simulink model "v01_aa_rii_16r4t11f_ver124sa"  (Simulink 7.6)
settings: Solver=ode45  StartTime=0.0  StopTime=128000  RelTol=1e-3
undefined symbols (not defined in the file or in its folder): sfp
libraries: xbsIndex_r4 x27287, monroe_library x154, xbsReplacements_r4 x78, casper_library_misc x33, simulink x32, dspsigops x32, xps_library x24
(part 4 of 178)

== system v01_aa_rii_16r4t11f_ver124sa/cram4 ==
  In1 : Inport
  In2 : Inport  Port=2
  In3 : Inport  Port=3
  In4 : Inport  Port=4
  In5 : Inport  Port=5
  In6 : Inport  Port=6
  In7 : Inport  Port=7
  In8 : Inport  Port=8
  In9 : Inport  Port=9
  In10 : Inport  Port=10
  In11 : Inport  Port=11
  In12 : Inport  Port=12
  In13 : Inport  Port=13
  In14 : Inport  Port=14
  In15 : Inport  Port=15
  In16 : Inport  Port=16
  In17 : Inport  Port=17
  In18 : Inport  Port=18
  In19 : Inport  Port=19
  In20 : Inport  Port=20
  In21 : Inport  Port=21
  In22 : Inport  Port=22
  In23 : Inport  Port=23
  In24 : Inport  Port=24
  In25 : Inport  Port=25
  In26 : Inport  Port=26
  In27 : Inport  Port=27
  In28 : Inport  Port=28
  In29 : Inport  Port=29
  In30 : Inport  Port=30
  In31 : Inport  Port=31
  In32 : Inport  Port=32
  Concat : xbsIndex_r4/Concat  num_inputs=32  dbl_ovrd=off  block_type=concat
      settings: block_version=10.1.2
  Reinterp{1..32} (32 alike) : xbsIndex_r4/Reinterpret  force_arith_type=on  arith_type=Unsigned  force_bin_pt=on  bin_pt=0  block_type=reinterpret
      Reinterp1 settings: infoedit="Changes signal type without altering the binary representation.   You can changed the signal between signed and unsigned, and relocate the binary point.<P><P>Hardware notes: In hardware this block costs nothing.<P><P>Example:  Suppose the input is 6 bits wide, signed, with 2 fractional bits, and the output is forced to unsigned with 0 fractional bits.  Then an input of -2.0 (1110.00 in binary 2's complement) becomes an output of 56 (111000 in binary)."  block_version=10.1.2
      Reinterp10 settings: infoedit="Changes signal type without altering the binary representation.   You can changed the signal between signed and unsigned, and relocate the binary point.<br><br>Hardware notes: In hardware this block costs nothing.<br><br>Example:  Suppose the input is 6 bits wide, signed, with 2 fractional bits, and the output is forced to unsigned with 0 fractional bits.  Then an input of -2.0 (1110.00 in binary 2's complement) becomes an output of 56 (111000 in binary)."
      Reinterp11 settings: infoedit="Changes signal type without altering the binary representation.   You can changed the signal between signed and unsigned, and relocate the binary point.<br><br>Hardware notes: In hardware this block costs nothing.<br><br>Example:  Suppose the input is 6 bits wide, signed, with 2 fractional bits, and the output is forced to unsigned with 0 fractional bits.  Then an input of -2.0 (1110.00 in binary 2's complement) becomes an output of 56 (111000 in binary)."
      Reinterp12 settings: infoedit="Changes signal type without altering the binary representation.   You can changed the signal between signed and unsigned, and relocate the binary point.<br><br>Hardware notes: In hardware this block costs nothing.<br><br>Example:  Suppose the input is 6 bits wide, signed, with 2 fractional bits, and the output is forced to unsigned with 0 fractional bits.  Then an input of -2.0 (1110.00 in binary 2's complement) becomes an output of 56 (111000 in binary)."
      Reinterp13 settings: infoedit="Changes signal type without altering the binary representation.   You can changed the signal between signed and unsigned, and relocate the binary point.<br><br>Hardware notes: In hardware this block costs nothing.<br><br>Example:  Suppose the input is 6 bits wide, signed, with 2 fractional bits, and the output is forced to unsigned with 0 fractional bits.  Then an input of -2.0 (1110.00 in binary 2's complement) becomes an output of 56 (111000 in binary)."
      Reinterp14 settings: infoedit="Changes signal type without altering the binary representation.   You can changed the signal between signed and unsigned, and relocate the binary point.<br><br>Hardware notes: In hardware this block costs nothing.<br><br>Example:  Suppose the input is 6 bits wide, signed, with 2 fractional bits, and the output is forced to unsigned with 0 fractional bits.  Then an input of -2.0 (1110.00 in binary 2's complement) becomes an output of 56 (111000 in binary)."
      Reinterp15 settings: infoedit="Changes signal type without altering the binary representation.   You can changed the signal between signed and unsigned, and relocate the binary point.<br><br>Hardware notes: In hardware this block costs nothing.<br><br>Example:  Suppose the input is 6 bits wide, signed, with 2 fractional bits, and the output is forced to unsigned with 0 fractional bits.  Then an input of -2.0 (1110.00 in binary 2's complement) becomes an output of 56 (111000 in binary)."
      Reinterp16 settings: infoedit="Changes signal type without altering the binary representation.   You can changed the signal between signed and unsigned, and relocate the binary point.<br><br>Hardware notes: In hardware this block costs nothing.<br><br>Example:  Suppose the input is 6 bits wide, signed, with 2 fractional bits, and the output is forced to unsigned with 0 fractional bits.  Then an input of -2.0 (1110.00 in binary 2's complement) becomes an output of 56 (111000 in binary)."
      Reinterp2 settings: infoedit="Changes signal type without altering the binary representation.   You can changed the signal between signed and unsigned, and relocate the binary point.<P><P>Hardware notes: In hardware this block costs nothing.<P><P>Example:  Suppose the input is 6 bits wide, signed, with 2 fractional bits, and the output is forced to unsigned with 0 fractional bits.  Then an input of -2.0 (1110.00 in binary 2's complement) becomes an output of 56 (111000 in binary)."  block_version=10.1.2
      Reinterp3 settings: infoedit="Changes signal type without altering the binary representation.   You can changed the signal between signed and unsigned, and relocate the binary point.<br><br>Hardware notes: In hardware this block costs nothing.<br><br>Example:  Suppose the input is 6 bits wide, signed, with 2 fractional bits, and the output is forced to unsigned with 0 fractional bits.  Then an input of -2.0 (1110.00 in binary 2's complement) becomes an output of 56 (111000 in binary)."
      Reinterp4 settings: infoedit="Changes signal type without altering the binary representation.   You can changed the signal between signed and unsigned, and relocate the binary point.<br><br>Hardware notes: In hardware this block costs nothing.<br><br>Example:  Suppose the input is 6 bits wide, signed, with 2 fractional bits, and the output is forced to unsigned with 0 fractional bits.  Then an input of -2.0 (1110.00 in binary 2's complement) becomes an output of 56 (111000 in binary)."
      Reinterp5 settings: infoedit="Changes signal type without altering the binary representation.   You can changed the signal between signed and unsigned, and relocate the binary point.<br><br>Hardware notes: In hardware this block costs nothing.<br><br>Example:  Suppose the input is 6 bits wide, signed, with 2 fractional bits, and the output is forced to unsigned with 0 fractional bits.  Then an input of -2.0 (1110.00 in binary 2's complement) becomes an output of 56 (111000 in binary)."
      Reinterp6 settings: infoedit="Changes signal type without altering the binary representation.   You can changed the signal between signed and unsigned, and relocate the binary point.<br><br>Hardware notes: In hardware this block costs nothing.<br><br>Example:  Suppose the input is 6 bits wide, signed, with 2 fractional bits, and the output is forced to unsigned with 0 fractional bits.  Then an input of -2.0 (1110.00 in binary 2's complement) becomes an output of 56 (111000 in binary)."
      Reinterp7 settings: infoedit="Changes signal type without altering the binary representation.   You can changed the signal between signed and unsigned, and relocate the binary point.<br><br>Hardware notes: In hardware this block costs nothing.<br><br>Example:  Suppose the input is 6 bits wide, signed, with 2 fractional bits, and the output is forced to unsigned with 0 fractional bits.  Then an input of -2.0 (1110.00 in binary 2's complement) becomes an output of 56 (111000 in binary)."
      Reinterp8 settings: infoedit="Changes signal type without altering the binary representation.   You can changed the signal between signed and unsigned, and relocate the binary point.<br><br>Hardware notes: In hardware this block costs nothing.<br><br>Example:  Suppose the input is 6 bits wide, signed, with 2 fractional bits, and the output is forced to unsigned with 0 fractional bits.  Then an input of -2.0 (1110.00 in binary 2's complement) becomes an output of 56 (111000 in binary)."
      Reinterp9 settings: infoedit="Changes signal type without altering the binary representation.   You can changed the signal between signed and unsigned, and relocate the binary point.<br><br>Hardware notes: In hardware this block costs nothing.<br><br>Example:  Suppose the input is 6 bits wide, signed, with 2 fractional bits, and the output is forced to unsigned with 0 fractional bits.  Then an input of -2.0 (1110.00 in binary 2's complement) becomes an output of 56 (111000 in binary)."
  Out : Outport
  wires:
  Concat/1 -> Out/1
  In1/1 -> Reinterp1/1
  Reinterp1/1 -> Concat/1
  In2/1 -> Reinterp2/1
  Reinterp2/1 -> Concat/2
  In3/1 -> Reinterp3/1
  Reinterp3/1 -> Concat/3
  In4/1 -> Reinterp4/1
  Reinterp4/1 -> Concat/4
  In5/1 -> Reinterp5/1
  Reinterp5/1 -> Concat/5
  In6/1 -> Reinterp6/1
  Reinterp6/1 -> Concat/6
  In7/1 -> Reinterp7/1
  Reinterp7/1 -> Concat/7
  In8/1 -> Reinterp8/1
  Reinterp8/1 -> Concat/8
  In9/1 -> Reinterp9/1
  Reinterp9/1 -> Concat/9
  In10/1 -> Reinterp10/1
  Reinterp10/1 -> Concat/10
  In11/1 -> Reinterp11/1
  Reinterp11/1 -> Concat/11
  In12/1 -> Reinterp12/1
  Reinterp12/1 -> Concat/12
  In13/1 -> Reinterp13/1
  Reinterp13/1 -> Concat/13
  In14/1 -> Reinterp14/1
  Reinterp14/1 -> Concat/14
  In15/1 -> Reinterp15/1
  Reinterp15/1 -> Concat/15
  In16/1 -> Reinterp16/1
  Reinterp16/1 -> Concat/16
  In17/1 -> Reinterp17/1
  Reinterp17/1 -> Concat/17
  In18/1 -> Reinterp18/1
  Reinterp18/1 -> Concat/18
  In19/1 -> Reinterp19/1
  Reinterp19/1 -> Concat/19
  In20/1 -> Reinterp20/1
  Reinterp20/1 -> Concat/20
  In21/1 -> Reinterp21/1
  Reinterp21/1 -> Concat/21
  In22/1 -> Reinterp22/1
  Reinterp22/1 -> Concat/22
  In23/1 -> Reinterp23/1
  Reinterp23/1 -> Concat/23
  In24/1 -> Reinterp24/1
  Reinterp24/1 -> Concat/24
  In25/1 -> Reinterp25/1
  Reinterp25/1 -> Concat/25
  In26/1 -> Reinterp26/1
  Reinterp26/1 -> Concat/26
  In27/1 -> Reinterp27/1
  Reinterp27/1 -> Concat/27
  In28/1 -> Reinterp28/1
  Reinterp28/1 -> Concat/28
  In29/1 -> Reinterp29/1
  Reinterp29/1 -> Concat/29
  In30/1 -> Reinterp30/1
  Reinterp30/1 -> Concat/30
  In31/1 -> Reinterp31/1
  Reinterp31/1 -> Concat/31
  In32/1 -> Reinterp32/1
  Reinterp32/1 -> Concat/32

== system v01_aa_rii_16r4t11f_ver124sa/debug ==
  note: Generate stop
      | when in circular
      | capture mode
  note: Delay start
      | of capture
  note: ctrl:
      | bit0: Put a posedge here
      |         to enable a new capture.
      | bit1: ignore external trigger
      |         and trigger immediately.
      | bit2: ignore external write
      |         enable and capture on
      |         every FPGA clock.
      | bit3: continuously capture
      |         data until we get an
      |         external stop command.
  note: Offset in vector
      | from first element
      | captured, used
      | during circular capture
  note: Number of
      | bytes in
      | buffer and
      | done bit
  note: Number of valid
      | data samples to
      | skip after trigger
      | before starting
      | capture
  note: Value on vin
      | aligned with
      | first sample on
      | din captured
  din : Inport
  we : Inport  Port=2
  trig : Inport  Port=3
  add_del : xbsIndex_r4/Delay  rst=off  infoeditControl=Selection of Reset will increase slice count due to use of real FFs and instead of SRLs  en=off  latency=1  dbl_ovrd=off  reg_retiming=off  block_type=delay
  add_gen : Subsystem  AncestorBlock=casper_library_scopes/snapshot/add_gen  mask{counter_size=14+2+1, increment=4, nsamples=14, burst_size=0, use_dsp48=on}
      mask prompts: counter_size "Address counter size (2^?)"; increment "Address counter increment"; nsamples "Number of samples (2^?)"; burst_size "Burst size (2^?)"; use_dsp48 "dsp48 counter/s"
      mask init:
      | set_param(gcb,'LinkStatus','inactive');
      | if strcmp(use_dsp48,'on'),
      |     set_param([gcb,'/add_gen'],'implementation','DSP48');
      | else
      |     set_param([gcb,'/add_gen'],'implementation','Fabric');
      | end
  basic_ctrl : Subsystem  AncestorBlock=casper_library_scopes/snapshot/basic_ctrl  mask{dram=off, data_width=32}
      mask prompts: data_width "data width"
      mask init:
      | set_param(gcb,'LinkStatus','inactive');
      | set_param([gcb,'/dram_munge'], 'dram', dram);
  bram : Subsystem  Tag=xps:bram  AncestorBlock=xps_library/Shared BRAM  mask{arith_type=Unsigned, addr_width=14, data_width=32, data_bin_pt=0, init_vals=[0:2^10-1], sample_rate=1}
      mask prompts: arith_type "Output Data Type"; addr_width "Address width"; data_width "Data Width"; data_bin_pt "Data Binary Point"; init_vals "Initial 32-bit values (simulation only)"; sample_rate "Sample rate"
      mask init:
      | shared_bram_mask;
      mask StyleString: popup(Unsigned|Signed  (2's comp)),edit,popup(8|16|32|64|128),edit,edit,edit
  cast : xbsIndex_r4/Convert  gui_display_data_type=Fixed-point  arith_type=Unsigned  n_bits=32  bin_pt=0  float_type=Single  exp_bits=8  fraction_bits=24  quantization=Truncate  overflow=Wrap  en=off  latency=0  dbl_ovrd=off  pipeline=off  block_type=convert
  const0 : Constant  Value=0
  ctrl : xps_library/software register  Tag=xps:sw_reg  SystemSampleTime=-1  io_dir=From Processor  arith_type=Unsigned  bitwidth=32  bin_pt=0  sample_period=1  gw_name=v01_aa_rii_16r4t11f_ver122sa_debug_ctrl_user_data_out
  dat_del : xbsIndex_r4/Delay  rst=off  infoeditControl=Selection of Reset will increase slice count due to use of real FFs and instead of SRLs  en=off  latency=1  dbl_ovrd=off  reg_retiming=off  block_type=delay
  g_tr_en_cnt : Terminator
  gbram : Terminator
  gstatus : Terminator
  gval : Terminator
  never : xbsIndex_r4/Constant  const=0  gui_display_data_type=Fixed-point  arith_type=Boolean  n_bits=16  bin_pt=14  preci_type=Single  exp_width=8  frac_width=24  explicit_period=on  period=1  equ=P=C  opselect=C  inp2=PCIN>>17  opr=+  inp1=P  carry=CIN  dbl_ovrd=off  block_type=constant
  ri : xbsIndex_r4/Reinterpret  force_arith_type=on  arith_type=Unsigned  force_bin_pt=on  bin_pt=0  block_type=reinterpret
  status : xps_library/software register  Tag=xps:sw_reg  SystemSampleTime=-1  io_dir=To Processor  arith_type=Unsigned  bitwidth=32  bin_pt=0  sample_period=1  gw_name=v01_aa_rii_16r4t11f_ver122sa_debug_status_user_data_in
  vin_const : xbsIndex_r4/Constant  const=0  gui_display_data_type=Fixed-point  arith_type=Boolean  n_bits=16  bin_pt=14  preci_type=Single  exp_width=8  frac_width=24  explicit_period=on  period=1  equ=P=C  opselect=C  inp2=PCIN>>17  opr=+  inp1=P  carry=CIN  dbl_ovrd=off  block_type=constant
  we_del : xbsIndex_r4/Delay  rst=off  infoeditControl=Selection of Reset will increase slice count due to use of real FFs and instead of SRLs  en=off  latency=1  dbl_ovrd=off  reg_retiming=off  block_type=delay
  wires:
  din/1 -> ri/1
  ri/1 -> cast/1
  cast/1 -> basic_ctrl/2(din)
  we/1 -> basic_ctrl/3(we)
  trig/1 -> basic_ctrl/4(trig)
  never/1 -> basic_ctrl/6(stopi)
  const0/1 -> ctrl/1
  ctrl/1 -> basic_ctrl/5(ctrl)
  basic_ctrl/1(vout) -> add_gen/1(vin)
  basic_ctrl/2(dout) -> add_gen/2(din)
  basic_ctrl/3(we_o) -> add_gen/3(we)
  basic_ctrl/4(go) -> add_gen/4(go)
  basic_ctrl/5(stop) -> add_gen/5(cont)
  basic_ctrl/6(init) -> add_gen/6(init)
  add_gen/5(status) -> status/1
  status/1 -> gstatus/1
  vin_const/1 -> basic_ctrl/1(vin)
  add_gen/1(vout) -> gval/1
  add_gen/6(en_cnt) -> g_tr_en_cnt/1
  add_gen/2(add) -> add_del/1
  add_gen/3(dout) -> dat_del/1
  add_gen/4(we_o) -> we_del/1
  add_del/1 -> bram/1(addr)
  dat_del/1 -> bram/2(data_in)
  we_del/1 -> bram/3(we)
  bram/1(data_out) -> gbram/1

== system v01_aa_rii_16r4t11f_ver124sa/fft_wideband_real ==
  sync : Inport
  shift : Inport  Port=2
  din_0 : Inport  Port=3
  din_1 : Inport  Port=4
  din_2 : Inport  Port=5
  din_3 : Inport  Port=6
  din_4 : Inport  Port=7
  din_5 : Inport  Port=8
  din_6 : Inport  Port=9
  din_7 : Inport  Port=10
  din_8 : Inport  Port=11
  din_9 : Inport  Port=12
  din_10 : Inport  Port=13
  din_11 : Inport  Port=14
  din_12 : Inport  Port=15
  din_13 : Inport  Port=16
  din_14 : Inport  Port=17
  din_15 : Inport  Port=18
  fft_biplex_real_4x0 : Subsystem
      settings: AttributesFormatString=|
      | Virtex5
      | 7 stages
      | [18,18]
      | Truncate
      | Wrap
      | 0 0 0 0 0 0 0 0 0 0 0 0 0 0 0 0 0 0 0
  fft_biplex_real_4x1 : Subsystem
      settings: AttributesFormatString=|
      | Virtex5
      | 7 stages
      | [18,18]
      | Truncate
      | Wrap
      | 0 0 0 0 0 0 0 0 0 0 0 0 0 0 0 0 0 0 0
  fft_biplex_real_4x2 : Subsystem
      settings: AttributesFormatString=|
      | Virtex5
      | 7 stages
      | [18,18]
      | Truncate
      | Wrap
      | 0 0 0 0 0 0 0 0 0 0 0 0 0 0 0 0 0 0 0
  fft_biplex_real_4x3 : Subsystem
      settings: AttributesFormatString=|
      | Virtex5
      | 7 stages
      | [18,18]
      | Truncate
      | Wrap
      | 0 0 0 0 0 0 0 0 0 0 0 0 0 0 0 0 0 0 0
  fft_direct : Subsystem
      settings: AttributesFormatString=|
      | Virtex5
      | stages [8   9  10  11] of 11
      | [18,18]
      | Truncate
      | Wrap
      | 0 0 0 0 0 0 0 0 0
  fft_unscrambler : Subsystem
      settings: AttributesFormatString=FFTSize=10, n_inputs=3
  in_del_4x0pol1 : Subsystem
      settings: AttributesFormatString=Latency = 0
  in_del_4x0pol2 : Subsystem
      settings: AttributesFormatString=Latency = 0
  in_del_4x0pol3 : Subsystem
      settings: AttributesFormatString=Latency = 0
  in_del_4x0pol4 : Subsystem
      settings: AttributesFormatString=Latency = 0
  in_del_4x1pol1 : Subsystem
      settings: AttributesFormatString=Latency = 0
  in_del_4x1pol2 : Subsystem
      settings: AttributesFormatString=Latency = 0
  in_del_4x1pol3 : Subsystem
      settings: AttributesFormatString=Latency = 0
  in_del_4x1pol4 : Subsystem
      settings: AttributesFormatString=Latency = 0
  in_del_4x2pol1 : Subsystem
      settings: AttributesFormatString=Latency = 0
  in_del_4x2pol2 : Subsystem
      settings: AttributesFormatString=Latency = 0
  in_del_4x2pol3 : Subsystem
      settings: AttributesFormatString=Latency = 0
  in_del_4x2pol4 : Subsystem
      settings: AttributesFormatString=Latency = 0
  in_del_4x3pol1 : Subsystem
      settings: AttributesFormatString=Latency = 0
  in_del_4x3pol2 : Subsystem
      settings: AttributesFormatString=Latency = 0
  in_del_4x3pol3 : Subsystem
      settings: AttributesFormatString=Latency = 0
  in_del_4x3pol4 : Subsystem
      settings: AttributesFormatString=Latency = 0
  in_del_sync_4x0 : Subsystem
      settings: AttributesFormatString=Latency = 0
  in_del_sync_4x1 : Subsystem
      settings: AttributesFormatString=Latency = 0
  in_del_sync_4x2 : Subsystem
      settings: AttributesFormatString=Latency = 0
  in_del_sync_4x3 : Subsystem
      settings: AttributesFormatString=Latency = 0
  of_det : xbsIndex_r4/Logical  logical_function=OR  inputs=5  en=off  latency=1  precision=Full  arith_type=Unsigned  n_bits=16  bin_pt=0  align_bp=on  dbl_ovrd=off  block_type=logical
  out_del_4x0pol1 : Subsystem
      settings: AttributesFormatString=Latency = 0
  out_del_4x0pol2 : Subsystem
      settings: AttributesFormatString=Latency = 0
  out_del_4x0pol3 : Subsystem
      settings: AttributesFormatString=Latency = 0
  out_del_4x0pol4 : Subsystem
      settings: AttributesFormatString=Latency = 0
  out_del_4x1pol1 : Subsystem
      settings: AttributesFormatString=Latency = 0
  out_del_4x1pol2 : Subsystem
      settings: AttributesFormatString=Latency = 0
  out_del_4x1pol3 : Subsystem
      settings: AttributesFormatString=Latency = 0
  out_del_4x1pol4 : Subsystem
      settings: AttributesFormatString=Latency = 0
  out_del_4x2pol1 : Subsystem
      settings: AttributesFormatString=Latency = 0
  out_del_4x2pol2 : Subsystem
      settings: AttributesFormatString=Latency = 0
  out_del_4x2pol3 : Subsystem
      settings: AttributesFormatString=Latency = 0
  out_del_4x2pol4 : Subsystem
      settings: AttributesFormatString=Latency = 0
  out_del_4x3pol1 : Subsystem
      settings: AttributesFormatString=Latency = 0
  out_del_4x3pol2 : Subsystem
      settings: AttributesFormatString=Latency = 0
  out_del_4x3pol3 : Subsystem
      settings: AttributesFormatString=Latency = 0
  out_del_4x3pol4 : Subsystem
      settings: AttributesFormatString=Latency = 0
  out_del_sync_4x0 : Subsystem
      settings: AttributesFormatString=Latency = 0
  shift_slice : xbsIndex_r4/Slice  nbits=4  boolean_output=off  mode=Lower Bit Location + Width  bit1=0  base1=MSB of Input  bit0=7  base0=LSB of Input  dbl_ovrd=off  block_type=slice
  sync_out : Outport
  dout0 : Outport  Port=2
  dout1 : Outport  Port=3
  dout2 : Outport  Port=4
  dout3 : Outport  Port=5
  dout4 : Outport  Port=6
  dout5 : Outport  Port=7
  dout6 : Outport  Port=8
  dout7 : Outport  Port=9
  of : Outport  Port=10
  wires:
  shift/1 -> fft_biplex_real_4x3/2(shift), fft_biplex_real_4x2/2(shift), fft_biplex_real_4x1/2(shift), fft_biplex_real_4x0/2(shift), shift_slice/1
  sync/1 -> in_del_sync_4x3/1(d), in_del_sync_4x2/1(d), in_del_sync_4x1/1(d), in_del_sync_4x0/1(d)
  of_det/1 -> of/1
  fft_direct/18(of) -> of_det/5
  fft_biplex_real_4x3/6(of) -> of_det/4
  fft_biplex_real_4x2/6(of) -> of_det/3
  fft_biplex_real_4x1/6(of) -> of_det/2
  fft_biplex_real_4x0/6(of) -> of_det/1
  fft_unscrambler/9(Out8) -> dout7/1
  fft_unscrambler/8(Out7) -> dout6/1
  fft_unscrambler/7(Out6) -> dout5/1
  fft_unscrambler/6(Out5) -> dout4/1
  fft_unscrambler/5(Out4) -> dout3/1
  fft_unscrambler/4(Out3) -> dout2/1
  fft_unscrambler/3(Out2) -> dout1/1
  fft_unscrambler/2(Out1) -> dout0/1
  fft_unscrambler/1(sync_out) -> sync_out/1
  fft_direct/9(dout_7) -> fft_unscrambler/9(In8)
  fft_direct/8(dout_6) -> fft_unscrambler/8(In7)
  fft_direct/7(dout_5) -> fft_unscrambler/7(In6)
  fft_direct/6(dout_4) -> fft_unscrambler/6(In5)
  fft_direct/5(dout_3) -> fft_unscrambler/5(In4)
  fft_direct/4(dout_2) -> fft_unscrambler/4(In3)
  fft_direct/3(dout_1) -> fft_unscrambler/3(In2)
  fft_direct/2(dout_0) -> fft_unscrambler/2(In1)
  fft_direct/1(sync_out) -> fft_unscrambler/1(sync)
  out_del_4x3pol4/1(q) -> fft_direct/18(din_15)
  out_del_4x3pol3/1(q) -> fft_direct/17(din_14)
  out_del_4x3pol2/1(q) -> fft_direct/16(din_13)
  out_del_4x3pol1/1(q) -> fft_direct/15(din_12)
  out_del_4x2pol4/1(q) -> fft_direct/14(din_11)
  out_del_4x2pol3/1(q) -> fft_direct/13(din_10)
  out_del_4x2pol2/1(q) -> fft_direct/12(din_9)
  out_del_4x2pol1/1(q) -> fft_direct/11(din_8)
  out_del_4x1pol4/1(q) -> fft_direct/10(din_7)
  out_del_4x1pol3/1(q) -> fft_direct/9(din_6)
  out_del_4x1pol2/1(q) -> fft_direct/8(din_5)
  out_del_4x1pol1/1(q) -> fft_direct/7(din_4)
  out_del_4x0pol4/1(q) -> fft_direct/6(din_3)
  out_del_4x0pol3/1(q) -> fft_direct/5(din_2)
  out_del_4x0pol2/1(q) -> fft_direct/4(din_1)
  out_del_4x0pol1/1(q) -> fft_direct/3(din_0)
  shift_slice/1 -> fft_direct/2(shift)
  out_del_sync_4x0/1(q) -> fft_direct/1(sync)
  fft_biplex_real_4x3/5(pol4_out) -> out_del_4x3pol4/1(d)
  fft_biplex_real_4x3/4(pol3_out) -> out_del_4x3pol3/1(d)
  fft_biplex_real_4x3/3(pol2_out) -> out_del_4x3pol2/1(d)
  fft_biplex_real_4x3/2(pol1_out) -> out_del_4x3pol1/1(d)
  in_del_4x3pol4/1(q) -> fft_biplex_real_4x3/6(pol4)
  in_del_4x3pol3/1(q) -> fft_biplex_real_4x3/5(pol3)
  in_del_4x3pol2/1(q) -> fft_biplex_real_4x3/4(pol2)
  in_del_4x3pol1/1(q) -> fft_biplex_real_4x3/3(pol1)
  in_del_sync_4x3/1(q) -> fft_biplex_real_4x3/1(sync)
  din_15/1 -> in_del_4x3pol4/1(d)
  din_14/1 -> in_del_4x3pol3/1(d)
  din_13/1 -> in_del_4x3pol2/1(d)
  din_12/1 -> in_del_4x3pol1/1(d)
  fft_biplex_real_4x2/5(pol4_out) -> out_del_4x2pol4/1(d)
  fft_biplex_real_4x2/4(pol3_out) -> out_del_4x2pol3/1(d)
  fft_biplex_real_4x2/3(pol2_out) -> out_del_4x2pol2/1(d)
  fft_biplex_real_4x2/2(pol1_out) -> out_del_4x2pol1/1(d)
  in_del_4x2pol4/1(q) -> fft_biplex_real_4x2/6(pol4)
  in_del_4x2pol3/1(q) -> fft_biplex_real_4x2/5(pol3)
  in_del_4x2pol2/1(q) -> fft_biplex_real_4x2/4(pol2)
  in_del_4x2pol1/1(q) -> fft_biplex_real_4x2/3(pol1)
  in_del_sync_4x2/1(q) -> fft_biplex_real_4x2/1(sync)
  din_11/1 -> in_del_4x2pol4/1(d)
  din_10/1 -> in_del_4x2pol3/1(d)
  din_9/1 -> in_del_4x2pol2/1(d)
  din_8/1 -> in_del_4x2pol1/1(d)
  fft_biplex_real_4x1/5(pol4_out) -> out_del_4x1pol4/1(d)
  fft_biplex_real_4x1/4(pol3_out) -> out_del_4x1pol3/1(d)
  fft_biplex_real_4x1/3(pol2_out) -> out_del_4x1pol2/1(d)
  fft_biplex_real_4x1/2(pol1_out) -> out_del_4x1pol1/1(d)
  in_del_4x1pol4/1(q) -> fft_biplex_real_4x1/6(pol4)
  in_del_4x1pol3/1(q) -> fft_biplex_real_4x1/5(pol3)
  in_del_4x1pol2/1(q) -> fft_biplex_real_4x1/4(pol2)
  in_del_4x1pol1/1(q) -> fft_biplex_real_4x1/3(pol1)
  in_del_sync_4x1/1(q) -> fft_biplex_real_4x1/1(sync)
  din_7/1 -> in_del_4x1pol4/1(d)
  din_6/1 -> in_del_4x1pol3/1(d)
  din_5/1 -> in_del_4x1pol2/1(d)
  din_4/1 -> in_del_4x1pol1/1(d)
  fft_biplex_real_4x0/1(sync_out) -> out_del_sync_4x0/1(d)
  fft_biplex_real_4x0/5(pol4_out) -> out_del_4x0pol4/1(d)
  fft_biplex_real_4x0/4(pol3_out) -> out_del_4x0pol3/1(d)
  fft_biplex_real_4x0/3(pol2_out) -> out_del_4x0pol2/1(d)
  fft_biplex_real_4x0/2(pol1_out) -> out_del_4x0pol1/1(d)
  in_del_4x0pol4/1(q) -> fft_biplex_real_4x0/6(pol4)
  in_del_4x0pol3/1(q) -> fft_biplex_real_4x0/5(pol3)
  in_del_4x0pol2/1(q) -> fft_biplex_real_4x0/4(pol2)
  in_del_4x0pol1/1(q) -> fft_biplex_real_4x0/3(pol1)
  in_del_sync_4x0/1(q) -> fft_biplex_real_4x0/1(sync)
  din_3/1 -> in_del_4x0pol4/1(d)
  din_2/1 -> in_del_4x0pol3/1(d)
  din_1/1 -> in_del_4x0pol2/1(d)
  din_0/1 -> in_del_4x0pol1/1(d)

== system v01_aa_rii_16r4t11f_ver124sa/fft_wideband_real1 ==
  sync : Inport
  shift : Inport  Port=2
  din_0 : Inport  Port=3
  din_1 : Inport  Port=4
  din_2 : Inport  Port=5
  din_3 : Inport  Port=6
  din_4 : Inport  Port=7
  din_5 : Inport  Port=8
  din_6 : Inport  Port=9
  din_7 : Inport  Port=10
  din_8 : Inport  Port=11
  din_9 : Inport  Port=12
  din_10 : Inport  Port=13
  din_11 : Inport  Port=14
  din_12 : Inport  Port=15
  din_13 : Inport  Port=16
  din_14 : Inport  Port=17
  din_15 : Inport  Port=18
  fft_biplex_real_4x0 : Subsystem
      settings: AttributesFormatString=|
      | Virtex5
      | 7 stages
      | [18,18]
      | Truncate
      | Wrap
      | 0 0 0 0 0 0 0 0 0 0 0 0 0 0 0 0 0 0 0
  fft_biplex_real_4x1 : Subsystem
      settings: AttributesFormatString=|
      | Virtex5
      | 7 stages
      | [18,18]
      | Truncate
      | Wrap
      | 0 0 0 0 0 0 0 0 0 0 0 0 0 0 0 0 0 0 0
  fft_biplex_real_4x2 : Subsystem
      settings: AttributesFormatString=|
      | Virtex5
      | 7 stages
      | [18,18]
      | Truncate
      | Wrap
      | 0 0 0 0 0 0 0 0 0 0 0 0 0 0 0 0 0 0 0
  fft_biplex_real_4x3 : Subsystem
      settings: AttributesFormatString=|
      | Virtex5
      | 7 stages
      | [18,18]
      | Truncate
      | Wrap
      | 0 0 0 0 0 0 0 0 0 0 0 0 0 0 0 0 0 0 0
  fft_direct : Subsystem
      settings: AttributesFormatString=|
      | Virtex5
      | stages [8   9  10  11] of 11
      | [18,18]
      | Truncate
      | Wrap
      | 0 0 0 0 0 0 0 0 0
  fft_unscrambler : Subsystem
      settings: AttributesFormatString=FFTSize=10, n_inputs=3
  in_del_4x0pol1 : Subsystem
      settings: AttributesFormatString=Latency = 0
  in_del_4x0pol2 : Subsystem
      settings: AttributesFormatString=Latency = 0
  in_del_4x0pol3 : Subsystem
      settings: AttributesFormatString=Latency = 0
  in_del_4x0pol4 : Subsystem
      settings: AttributesFormatString=Latency = 0
  in_del_4x1pol1 : Subsystem
      settings: AttributesFormatString=Latency = 0
  in_del_4x1pol2 : Subsystem
      settings: AttributesFormatString=Latency = 0
  in_del_4x1pol3 : Subsystem
      settings: AttributesFormatString=Latency = 0
  in_del_4x1pol4 : Subsystem
      settings: AttributesFormatString=Latency = 0
  in_del_4x2pol1 : Subsystem
      settings: AttributesFormatString=Latency = 0
  in_del_4x2pol2 : Subsystem
      settings: AttributesFormatString=Latency = 0
  in_del_4x2pol3 : Subsystem
      settings: AttributesFormatString=Latency = 0
  in_del_4x2pol4 : Subsystem
      settings: AttributesFormatString=Latency = 0
  in_del_4x3pol1 : Subsystem
      settings: AttributesFormatString=Latency = 0
  in_del_4x3pol2 : Subsystem
      settings: AttributesFormatString=Latency = 0
  in_del_4x3pol3 : Subsystem
      settings: AttributesFormatString=Latency = 0
  in_del_4x3pol4 : Subsystem
      settings: AttributesFormatString=Latency = 0
  in_del_sync_4x0 : Subsystem
      settings: AttributesFormatString=Latency = 0
  in_del_sync_4x1 : Subsystem
      settings: AttributesFormatString=Latency = 0
  in_del_sync_4x2 : Subsystem
      settings: AttributesFormatString=Latency = 0
  in_del_sync_4x3 : Subsystem
      settings: AttributesFormatString=Latency = 0
  of_det : xbsIndex_r4/Logical  logical_function=OR  inputs=5  en=off  latency=1  precision=Full  arith_type=Unsigned  n_bits=16  bin_pt=0  align_bp=on  dbl_ovrd=off  block_type=logical
  out_del_4x0pol1 : Subsystem
      settings: AttributesFormatString=Latency = 0
  out_del_4x0pol2 : Subsystem
      settings: AttributesFormatString=Latency = 0
  out_del_4x0pol3 : Subsystem
      settings: AttributesFormatString=Latency = 0
  out_del_4x0pol4 : Subsystem
      settings: AttributesFormatString=Latency = 0
  out_del_4x1pol1 : Subsystem
      settings: AttributesFormatString=Latency = 0
  out_del_4x1pol2 : Subsystem
      settings: AttributesFormatString=Latency = 0
  out_del_4x1pol3 : Subsystem
      settings: AttributesFormatString=Latency = 0
  out_del_4x1pol4 : Subsystem
      settings: AttributesFormatString=Latency = 0
  out_del_4x2pol1 : Subsystem
      settings: AttributesFormatString=Latency = 0
  out_del_4x2pol2 : Subsystem
      settings: AttributesFormatString=Latency = 0
  out_del_4x2pol3 : Subsystem
      settings: AttributesFormatString=Latency = 0
  out_del_4x2pol4 : Subsystem
      settings: AttributesFormatString=Latency = 0
  out_del_4x3pol1 : Subsystem
      settings: AttributesFormatString=Latency = 0
  out_del_4x3pol2 : Subsystem
      settings: AttributesFormatString=Latency = 0
  out_del_4x3pol3 : Subsystem
      settings: AttributesFormatString=Latency = 0
  out_del_4x3pol4 : Subsystem
      settings: AttributesFormatString=Latency = 0
  out_del_sync_4x0 : Subsystem
      settings: AttributesFormatString=Latency = 0
  shift_slice : xbsIndex_r4/Slice  nbits=4  boolean_output=off  mode=Lower Bit Location + Width  bit1=0  base1=MSB of Input  bit0=7  base0=LSB of Input  dbl_ovrd=off  block_type=slice
  sync_out : Outport
  dout0 : Outport  Port=2
  dout1 : Outport  Port=3
  dout2 : Outport  Port=4
  dout3 : Outport  Port=5
  dout4 : Outport  Port=6
  dout5 : Outport  Port=7
  dout6 : Outport  Port=8
  dout7 : Outport  Port=9
  of : Outport  Port=10
  wires:
  shift/1 -> fft_biplex_real_4x3/2(shift), fft_biplex_real_4x2/2(shift), fft_biplex_real_4x1/2(shift), fft_biplex_real_4x0/2(shift), shift_slice/1
  sync/1 -> in_del_sync_4x3/1(d), in_del_sync_4x2/1(d), in_del_sync_4x1/1(d), in_del_sync_4x0/1(d)
  of_det/1 -> of/1
  fft_direct/18(of) -> of_det/5
  fft_biplex_real_4x3/6(of) -> of_det/4
  fft_biplex_real_4x2/6(of) -> of_det/3
  fft_biplex_real_4x1/6(of) -> of_det/2
  fft_biplex_real_4x0/6(of) -> of_det/1
  fft_unscrambler/9(Out8) -> dout7/1
  fft_unscrambler/8(Out7) -> dout6/1
  fft_unscrambler/7(Out6) -> dout5/1
  fft_unscrambler/6(Out5) -> dout4/1
  fft_unscrambler/5(Out4) -> dout3/1
  fft_unscrambler/4(Out3) -> dout2/1
  fft_unscrambler/3(Out2) -> dout1/1
  fft_unscrambler/2(Out1) -> dout0/1
  fft_unscrambler/1(sync_out) -> sync_out/1
  fft_direct/9(dout_7) -> fft_unscrambler/9(In8)
  fft_direct/8(dout_6) -> fft_unscrambler/8(In7)
  fft_direct/7(dout_5) -> fft_unscrambler/7(In6)
  fft_direct/6(dout_4) -> fft_unscrambler/6(In5)
  fft_direct/5(dout_3) -> fft_unscrambler/5(In4)
  fft_direct/4(dout_2) -> fft_unscrambler/4(In3)
  fft_direct/3(dout_1) -> fft_unscrambler/3(In2)
  fft_direct/2(dout_0) -> fft_unscrambler/2(In1)
  fft_direct/1(sync_out) -> fft_unscrambler/1(sync)
  out_del_4x3pol4/1(q) -> fft_direct/18(din_15)
  out_del_4x3pol3/1(q) -> fft_direct/17(din_14)
  out_del_4x3pol2/1(q) -> fft_direct/16(din_13)
  out_del_4x3pol1/1(q) -> fft_direct/15(din_12)
  out_del_4x2pol4/1(q) -> fft_direct/14(din_11)
  out_del_4x2pol3/1(q) -> fft_direct/13(din_10)
  out_del_4x2pol2/1(q) -> fft_direct/12(din_9)
  out_del_4x2pol1/1(q) -> fft_direct/11(din_8)
  out_del_4x1pol4/1(q) -> fft_direct/10(din_7)
  out_del_4x1pol3/1(q) -> fft_direct/9(din_6)
  out_del_4x1pol2/1(q) -> fft_direct/8(din_5)
  out_del_4x1pol1/1(q) -> fft_direct/7(din_4)
  out_del_4x0pol4/1(q) -> fft_direct/6(din_3)
  out_del_4x0pol3/1(q) -> fft_direct/5(din_2)
  out_del_4x0pol2/1(q) -> fft_direct/4(din_1)
  out_del_4x0pol1/1(q) -> fft_direct/3(din_0)
  shift_slice/1 -> fft_direct/2(shift)
  out_del_sync_4x0/1(q) -> fft_direct/1(sync)
  fft_biplex_real_4x3/5(pol4_out) -> out_del_4x3pol4/1(d)
  fft_biplex_real_4x3/4(pol3_out) -> out_del_4x3pol3/1(d)
  fft_biplex_real_4x3/3(pol2_out) -> out_del_4x3pol2/1(d)
  fft_biplex_real_4x3/2(pol1_out) -> out_del_4x3pol1/1(d)
  in_del_4x3pol4/1(q) -> fft_biplex_real_4x3/6(pol4)
  in_del_4x3pol3/1(q) -> fft_biplex_real_4x3/5(pol3)
  in_del_4x3pol2/1(q) -> fft_biplex_real_4x3/4(pol2)
  in_del_4x3pol1/1(q) -> fft_biplex_real_4x3/3(pol1)
  in_del_sync_4x3/1(q) -> fft_biplex_real_4x3/1(sync)
  din_15/1 -> in_del_4x3pol4/1(d)
  din_14/1 -> in_del_4x3pol3/1(d)
  din_13/1 -> in_del_4x3pol2/1(d)
  din_12/1 -> in_del_4x3pol1/1(d)
  fft_biplex_real_4x2/5(pol4_out) -> out_del_4x2pol4/1(d)
  fft_biplex_real_4x2/4(pol3_out) -> out_del_4x2pol3/1(d)
  fft_biplex_real_4x2/3(pol2_out) -> out_del_4x2pol2/1(d)
  fft_biplex_real_4x2/2(pol1_out) -> out_del_4x2pol1/1(d)
  in_del_4x2pol4/1(q) -> fft_biplex_real_4x2/6(pol4)
  in_del_4x2pol3/1(q) -> fft_biplex_real_4x2/5(pol3)
  in_del_4x2pol2/1(q) -> fft_biplex_real_4x2/4(pol2)
  in_del_4x2pol1/1(q) -> fft_biplex_real_4x2/3(pol1)
  in_del_sync_4x2/1(q) -> fft_biplex_real_4x2/1(sync)
  din_11/1 -> in_del_4x2pol4/1(d)
  din_10/1 -> in_del_4x2pol3/1(d)
  din_9/1 -> in_del_4x2pol2/1(d)
  din_8/1 -> in_del_4x2pol1/1(d)
  fft_biplex_real_4x1/5(pol4_out) -> out_del_4x1pol4/1(d)
  fft_biplex_real_4x1/4(pol3_out) -> out_del_4x1pol3/1(d)
  fft_biplex_real_4x1/3(pol2_out) -> out_del_4x1pol2/1(d)
  fft_biplex_real_4x1/2(pol1_out) -> out_del_4x1pol1/1(d)
  in_del_4x1pol4/1(q) -> fft_biplex_real_4x1/6(pol4)
  in_del_4x1pol3/1(q) -> fft_biplex_real_4x1/5(pol3)
  in_del_4x1pol2/1(q) -> fft_biplex_real_4x1/4(pol2)
  in_del_4x1pol1/1(q) -> fft_biplex_real_4x1/3(pol1)
  in_del_sync_4x1/1(q) -> fft_biplex_real_4x1/1(sync)
  din_7/1 -> in_del_4x1pol4/1(d)
  din_6/1 -> in_del_4x1pol3/1(d)
  din_5/1 -> in_del_4x1pol2/1(d)
  din_4/1 -> in_del_4x1pol1/1(d)
  fft_biplex_real_4x0/1(sync_out) -> out_del_sync_4x0/1(d)
  fft_biplex_real_4x0/5(pol4_out) -> out_del_4x0pol4/1(d)
  fft_biplex_real_4x0/4(pol3_out) -> out_del_4x0pol3/1(d)
  fft_biplex_real_4x0/3(pol2_out) -> out_del_4x0pol2/1(d)
  fft_biplex_real_4x0/2(pol1_out) -> out_del_4x0pol1/1(d)
  in_del_4x0pol4/1(q) -> fft_biplex_real_4x0/6(pol4)
  in_del_4x0pol3/1(q) -> fft_biplex_real_4x0/5(pol3)
  in_del_4x0pol2/1(q) -> fft_biplex_real_4x0/4(pol2)
  in_del_4x0pol1/1(q) -> fft_biplex_real_4x0/3(pol1)
  in_del_sync_4x0/1(q) -> fft_biplex_real_4x0/1(sync)
  din_3/1 -> in_del_4x0pol4/1(d)
  din_2/1 -> in_del_4x0pol3/1(d)
  din_1/1 -> in_del_4x0pol2/1(d)
  din_0/1 -> in_del_4x0pol1/1(d)
